simulink library "Renault_Library"  (Simulink 8.2)
settings: Solver=ode3  StartTime=0.0  StopTime=10.0  FixedStep=0.020  RelTol=1e-3
undefined symbols (not defined in the file or in its folder): ABC, AssystemProposal, b, chkval, CoeffA_C, CoeffB_C, CoeffMean_C, ConfTime_C, DiagDelay_C, DividePercent, FailureConfActive_C, FallingEdgeDuration_C, HighLevelThresholdVoltage_C, HighLevelVoltage_C, HighLevelVoltageTunable_C, InitValue_C, InputVoltRangeMax_C, InputVoltRangeMin_C, LowActiveContact_C, LowLevelThresholdVoltage_C, LowLevelVoltage_C, MaxOperatingVoltage_C, MinOperatingVoltage_C, mV2V_conversion, OUT1, OutOfRangeHigh_C, OutOfRangeLow_C, PinSupplySaturationMax, PinSupplySaturationMin, Res1Value_C, Res2Value_C, Res3Value_C, RisingEdgeDuration_C, RSA_CANSignalFailMonitoring, SampleTime_C, service14Table, service19Table, service22Table, service24Table, service28Table, service2ETable, service2FTable, service31Table, service87Table, SizeXStateDID2FTable, SizeXTable14, SizeXTable19, SizeXTable22, SizeXTable24, SizeXTable28, SizeXTable2E, SizeXTable2F, SizeXTable31, SizeXTable87, SizeYStateDID2FTable, SizeYTable14, SizeYTable19, SizeYTable22, SizeYTable24, SizeYTable28 ... (+22)
undefined symbols (not defined in the file): BatteryVoltage_ActivationThreshold_C, FrameStatus_AbsentValue_C, FrameStatus_ConfAbsentValue_C, MemThreshold_C, NAMEOFTHEMODEL_SAMPLE_TIME_CST, NbAbs1_C, NbConfAbs1_C, NbConfPres1_C, NbPres1_C, NOMDUSIGNAL_FaultConfTime_C, NOMDUSIGNAL_FaultRehabTime_C, NOMDUSIGNAL_switchvalue_C, NOMDUSIGNAL_unavailablevalue_C, PeriodFrame1_C, ResetTime_C, WakeUpTime_C
libraries: Renault_Library x112, simulink x75
(part 15 of 32)

== system Renault_Library/Services ECU/Commonly_Used_Blocks/RSA_ServECU_EnaOutContactSat/SwitchWithoutSlope ==
  In1 : Inport
  Switch_Cmd : Inport  Port=2
  In0 : Inport  Port=3
  Enable : EnablePort  StatesWhenEnabling=reset
  Switch3 : Switch  Criteria=u2 ~= 0  RndMeth=Zero  InputSameDT=on
  Out1 : Outport  OutputWhenDisabled=reset  InitialOutput=0
  wires:
  Switch_Cmd/1 -> Switch3/2
  In1/1 -> Switch3/1
  Switch3/1 -> Out1/1
  In0/1 -> Switch3/3

== system Renault_Library/Services ECU/Commonly_Used_Blocks/RSA_ServECU_EnabledDiagnostic/DiagDelaySubsystem ==
  In1 : Inport
  DataTypeConversion1 : DataTypeConversion  RndMeth=Zero
      settings: AttributesFormatString=Saturation:%<SaturateOnIntegerOverflow>
  DiagDelay : Constant  Value=DiagDelay_C  OutDataTypeStr=Inherit: Inherit via back propagation  SampleTime=-1
  RSA_TurnOffDelay2 : Renault_Library/Complex blocks/Timers & Counters/RSA_TurnOffDelay2  Tag=[LIB]  Ts=SampleTime_C
  RSA_TurnOnDelay2 : Renault_Library/Complex blocks/Timers & Counters/RSA_TurnOnDelay2  Tag=[LIB]  Ts=SampleTime_C
  Terminator1 : Terminator
  Terminator2 : Terminator
  ms2m : Gain  Gain=.001  RndMeth=Zero
      settings: AttributesFormatString=Saturation:%<SaturateOnIntegerOverflow>
  Out1 : Outport
  wires:
  RSA_TurnOnDelay2/1 -> RSA_TurnOffDelay2/1
  DiagDelay/1 -> ms2m/1
  RSA_TurnOnDelay2/2 -> Terminator1/1
  In1/1 -> DataTypeConversion1/1
  RSA_TurnOffDelay2/1 -> Out1/1
  RSA_TurnOffDelay2/2 -> Terminator2/1
  DataTypeConversion1/1 -> RSA_TurnOnDelay2/1
  ms2m/1 -> RSA_TurnOffDelay2/2, RSA_TurnOnDelay2/2

== system Renault_Library/Services ECU/Commonly_Used_Blocks/RSA_ServECU_EnabledSupply/Mean ==
  Output : Inport
  CoeffB2 : Constant  Value=CoeffMean_C  OutDataTypeStr=Inherit: Inherit via back propagation  SampleTime=-1
  Moy_1 : Subsystem
  Moy_10 : Subsystem
  Moy_2 : Subsystem
  Moy_3 : Subsystem
  Moy_4 : Subsystem
  Moy_5 : Subsystem
  Moy_6 : Subsystem
  Moy_7 : Subsystem
  Moy_8 : Subsystem
  Moy_9 : Subsystem
  MultiportSwitch : MultiPortSwitch  Inputs=10  RndMeth=Zero  InputSameDT=on
  MeanOutputVoltage : Outport
  wires:
  Moy_1/1(Out1) -> MultiportSwitch/2
  Moy_2/1(Out1) -> MultiportSwitch/3
  Moy_3/1(Out1) -> MultiportSwitch/4
  Moy_4/1(Out1) -> MultiportSwitch/5
  Moy_5/1(Out1) -> MultiportSwitch/6
  Moy_6/1(Out1) -> MultiportSwitch/7
  Moy_7/1(Out1) -> MultiportSwitch/8
  Moy_8/1(Out1) -> MultiportSwitch/9
  Moy_9/1(Out1) -> MultiportSwitch/10
  Moy_10/1(Out1) -> MultiportSwitch/11
  MultiportSwitch/1 -> MeanOutputVoltage/1
  CoeffB2/1 -> MultiportSwitch/1
  Output/1 -> Moy_1/1(In1), Moy_2/1(In1), Moy_3/1(In1), Moy_4/1(In1), Moy_5/1(In1), Moy_6/1(In1), Moy_7/1(In1), Moy_8/1(In1), Moy_9/1(In1), Moy_10/1(In1)

== system Renault_Library/Services ECU/Commonly_Used_Blocks/RSA_ServECU_FaultConfirmation/FailureMonitoring ==
  CurrentFaultState : Inport
  Failure : Inport  Port=2
  ConfTime : Constant  Value=ConfTime_C  OutDataTypeStr=Inherit: Inherit via back propagation  SampleTime=-1
  FixPtSum2 : Sum  OutDataTypeStr=Inherit: Inherit via back propagation  RndMeth=Zero  InputSameDT=on  LockScale=on
  FixPtSwitch2 : Switch  Criteria=u2 ~= 0  Threshold=0.5  OutDataTypeStr=Inherit: Inherit via back propagation  RndMeth=Zero  ZeroCross=off  InputSameDT=on
  Gain : Gain  Gain=.001  RndMeth=Zero
      settings: AttributesFormatString=Saturation:%<SaturateOnIntegerOverflow>
  Gain2 : Gain  Gain=0.001  RndMeth=Zero
      settings: AttributesFormatString=Saturation:%<SaturateOnIntegerOverflow>
  LogicalOperator1 : Logic  OutDataTypeStr=boolean
  Output1 : UnitDelay  SampleTime=-1
  RelationalOperator : RelationalOperator  Operator===  OutDataTypeStr=boolean  InputSameDT=on
  RelationalOperator1 : RelationalOperator  OutDataTypeStr=boolean  ZeroCross=off  InputSameDT=on
  Reset : Constant  Value=0  OutDataTypeStr=Inherit: Inherit via back propagation  SampleTime=-1
  SampleTime : Constant  Value=SampleTime_C  OutDataTypeStr=Inherit: Inherit via back propagation  SampleTime=-1
  Switch1 : Switch  Criteria=u2 ~= 0  Threshold=0.5  OutDataTypeStr=Inherit: Inherit via back propagation  RndMeth=Zero  SaturateOnIntegerOverflow=on  InputSameDT=on
  Switch4 : Switch  Criteria=u2 ~= 0  Threshold=0.5  RndMeth=Zero  InputSameDT=on
  UnitDelay : UnitDelay  SampleTime=-1
  UnitDelay1 : UnitDelay  InitialCondition=InitValue_C  SampleTime=-1
  false : Constant  Value=false  OutDataTypeStr=boolean  SampleTime=-1
  true : Constant  Value=true  OutDataTypeStr=boolean  SampleTime=-1
  ConfirmedFaultValue : Outport
  failure_flag : Outport  Port=2
  wires:
  LogicalOperator1/1 -> FixPtSwitch2/2
  RelationalOperator/1 -> LogicalOperator1/2
  [CurrentFaultState] -> RelationalOperator/1, UnitDelay/1
  UnitDelay/1 -> RelationalOperator/2
  Gain2/1 -> [SampleTime]
  Output1/1 -> FixPtSum2/1
  [SampleTime] -> FixPtSum2/2
  SampleTime/1 -> Gain2/1
  Reset/1 -> [Reset]
  CurrentFaultState/1 -> [CurrentFaultState], Switch1/1
  true/1 -> Switch4/1
  false/1 -> Switch4/3
  Switch4/1 -> Switch1/2, failure_flag/1
  [Reset] -> FixPtSwitch2/3
  Failure/1 -> LogicalOperator1/1
  FixPtSwitch2/1 -> RelationalOperator1/1, Output1/1
  FixPtSum2/1 -> FixPtSwitch2/1
  RelationalOperator1/1 -> Switch4/2
  UnitDelay1/1 -> Switch1/3
  Switch1/1 -> UnitDelay1/1, ConfirmedFaultValue/1
  ConfTime/1 -> Gain/1
  Gain/1 -> RelationalOperator1/2

== system Renault_Library/Services ECU/Diag/RSA_DiagnosticManagement/DIAGNOSTIC_ACTION_TOP ==
  DIAG_BUFFER_REQUEST : Inport  OutDataTypeStr=uint8  PortDimensions=4095
  NEGATIVE_RES_CODE : Inport  Port=2
  SUPPRESS_POS_RES : Inport  Port=3
  SEND_RES_MESSAGE : Inport  Port=4
  LIST_DID_SERVICE_22 : Inport  Port=5
  DIAG_INPUT : Inport  Port=6
  Diagnostic_Action : Stateflow chart  TreatAsAtomicUnit=on
  UnitDelay4 : UnitDelay  SampleTime=-1
  DIAG_OUTPUT : Outport
  DIAG_BUFFER_RESPONSE : Outport  Port=2  OutDataTypeStr=uint8  PortDimensions=4095
  RES_DATA_NUMBER : Outport  Port=3
  wires:
  SEND_RES_MESSAGE/1 -> Diagnostic_Action/4(SEND_RES_MESSAGE)
  NEGATIVE_RES_CODE/1 -> Diagnostic_Action/2(NEGATIVE_RES_CODE)
  LIST_DID_SERVICE_22/1 -> Diagnostic_Action/5(LIST_DID_SERVICE_22)
  Diagnostic_Action/2(DIAG_BUFFER_RESPONSE) -> DIAG_BUFFER_RESPONSE/1
  Diagnostic_Action/3(RES_DATA_NUMBER) -> UnitDelay4/1
  DIAG_BUFFER_REQUEST/1 -> Diagnostic_Action/1(DIAG_BUFFER_REQUEST)
  SUPPRESS_POS_RES/1 -> Diagnostic_Action/3(SUPPRESS_POS_RES)
  DIAG_INPUT/1 -> Diagnostic_Action/6(DIAG_INPUT)
  Diagnostic_Action/1(DIAG_OUTPUT) -> DIAG_OUTPUT/1
  UnitDelay4/1 -> RES_DATA_NUMBER/1

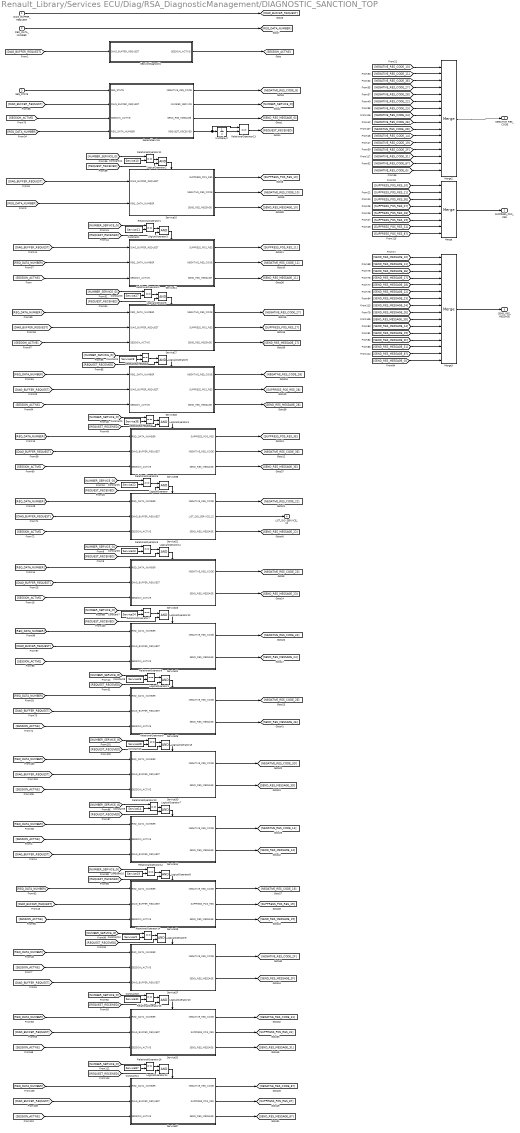
== system Renault_Library/Services ECU/Diag/RSA_DiagnosticManagement/DIAGNOSTIC_SANCTION_TOP ==
  DIAG_BUFFER_REQUEST : Inport  OutDataTypeStr=uint8  PortDimensions=4095
  REQ_DATA_NUMBER : Inport  Port=2  OutDataTypeStr=uint16
  SEG_STATE : Inport  Port=3  OutDataTypeStr=Enum: TYP_SEG_STATE
  Constant1 : Constant  Value=Service11  OutDataTypeStr=Inherit: Inherit via back propagation  SampleTime=-1
  Constant10 : Constant  Value=Service19  OutDataTypeStr=Inherit: Inherit via back propagation  SampleTime=-1
  Constant11 : Constant  Value=Service10  OutDataTypeStr=Inherit: Inherit via back propagation  SampleTime=-1
  Constant12 : Constant  Value=Service2F  OutDataTypeStr=Inherit: Inherit via back propagation  SampleTime=-1
  Constant13 : Constant  Value=Service3D  OutDataTypeStr=Inherit: Inherit via back propagation  SampleTime=-1
  Constant14 : Constant  Value=Service87  OutDataTypeStr=Inherit: Inherit via back propagation  SampleTime=-1
  Constant2 : Constant  Value=Service3E  OutDataTypeStr=Inherit: Inherit via back propagation  SampleTime=-1
  Constant20 : Constant  Value=Service31  OutDataTypeStr=Inherit: Inherit via back propagation  SampleTime=-1
  Constant3 : Constant  Value=Service27  OutDataTypeStr=Inherit: Inherit via back propagation  SampleTime=-1
  Constant4 : Constant  Value=Service28  OutDataTypeStr=Inherit: Inherit via back propagation  SampleTime=-1
  Constant5 : Constant  Value=Service22  OutDataTypeStr=Inherit: Inherit via back propagation  SampleTime=-1
  Constant6 : Constant  Value=Service23  OutDataTypeStr=Inherit: Inherit via back propagation  SampleTime=-1
  Constant7 : Constant  Value=Service24  OutDataTypeStr=Inherit: Inherit via back propagation  SampleTime=-1
  Constant8 : Constant  Value=Service2E  OutDataTypeStr=Inherit: Inherit via back propagation  SampleTime=-1
  Constant9 : Constant  Value=Service14  OutDataTypeStr=Inherit: Inherit via back propagation  SampleTime=-1
  GeneralService : Subsystem
  LogicalOperator{,1..14} (15 alike) : Logic  OutDataTypeStr=boolean
  Merge : Merge  Inputs=8
  Merge2 : Merge  Inputs=16
  Merge3 : Merge  Inputs=16
  RelationalOperator{1..16} (16 alike) : RelationalOperator  Operator===  OutDataTypeStr=boolean  InputSameDT=on
  Service10 : Subsystem  TreatAsAtomicUnit=on
  Service11 : Subsystem  TreatAsAtomicUnit=on
  Service14 : Subsystem  TreatAsAtomicUnit=on
  Service19 : Subsystem  TreatAsAtomicUnit=on
  Service22 : Subsystem  TreatAsAtomicUnit=on
  Service23 : Subsystem  TreatAsAtomicUnit=on
  Service24 : Subsystem  TreatAsAtomicUnit=on
  Service27 : Subsystem  TreatAsAtomicUnit=on
  Service28 : Subsystem  TreatAsAtomicUnit=on
  Service2E : Subsystem  TreatAsAtomicUnit=on
  Service2F : Subsystem  TreatAsAtomicUnit=on
  Service31 : Subsystem  TreatAsAtomicUnit=on
  Service3D : Subsystem  TreatAsAtomicUnit=on
  Service3E : Subsystem  TreatAsAtomicUnit=on
  Service87 : Subsystem  TreatAsAtomicUnit=on
  SessionDiagnostic : Subsystem
  UnitDelay2 : UnitDelay  SampleTime=-1
  NEGATIVE_RES_CODE : Outport
  SUPPRESS_POS_RES : Outport  Port=2
  SEND_RES_MESSAGE : Outport  Port=3
  LIST_DID_SERVICE_22 : Outport  Port=4  LockScale=on
  wires:
  [SESSION_ACTIVE] -> Service11/3(SESSION_ACTIVE)
  DIAG_BUFFER_REQUEST/1 -> [DIAG_BUFFER_REQUEST]
  REQ_DATA_NUMBER/1 -> [REQ_DATA_NUMBER]
  [DIAG_BUFFER_REQUEST] -> Service11/1(DIAG_BUFFER_REQUEST)
  [REQ_DATA_NUMBER] -> Service11/2(REQ_DATA_NUMBER)
  [REQ_DATA_NUMBER] -> Service14/1(REQ_DATA_NUMBER)
  SEG_STATE/1 -> GeneralService/1(SEG_STATE)
  [DIAG_BUFFER_REQUEST] -> GeneralService/2(DIAG_BUFFER_REQUEST)
  [SESSION_ACTIVE] -> GeneralService/3(SESSION_ACTIVE)
  GeneralService/2(NUMBER_SERVICE) -> [NUMBER_SERVICE_G]
  [NUMBER_SERVICE_G] -> RelationalOperator8/1
  Constant8/1 -> RelationalOperator8/2
  [REQ_DATA_NUMBER] -> Service2E/1(REQ_DATA_NUMBER)
  [REQ_DATA_NUMBER] -> Service19/1(REQ_DATA_NUMBER)
  [DIAG_BUFFER_REQUEST] -> Service19/2(DIAG_BUFFER_REQUEST)
  [SESSION_ACTIVE] -> Service19/3(SESSION_ACTIVE)
  [REQ_DATA_NUMBER] -> Service2F/1(REQ_DATA_NUMBER)
  [REQ_DATA_NUMBER] -> Service31/1(REQ_DATA_NUMBER)
  [DIAG_BUFFER_REQUEST] -> Service31/2(DIAG_BUFFER_REQUEST)
  [SESSION_ACTIVE] -> Service31/3(SESSION_ACTIVE)
  [DIAG_BUFFER_REQUEST] -> Service10/1(DIAG_BUFFER_REQUEST)
  [REQ_DATA_NUMBER] -> Service10/2(REQ_DATA_NUMBER)
  [DIAG_BUFFER_REQUEST] -> Service3E/2(DIAG_BUFFER_REQUEST)
  [REQ_DATA_NUMBER] -> Service3E/1(REQ_DATA_NUMBER)
  [SESSION_ACTIVE] -> Service3E/3(SESSION_ACTIVE)
  SessionDiagnostic/1(SESSION_ACTIVE) -> [SESSION_ACTIVE]
  [DIAG_BUFFER_REQUEST] -> SessionDiagnostic/1(DIAG_BUFFER_REQUEST)
  GeneralService/1(NEGATIVE_RES_CODE) -> [NEGATIVE_RES_CODE_G]
  Service10/1(SUPPRESS_POS_RES) -> [SUPPRESS_POS_RES_10]
  Service10/2(NEGATIVE_RES_CODE) -> [NEGATIVE_RES_CODE_10]
  Service11/1(SUPPRESS_POS_RES) -> [SUPPRESS_POS_RES_11]
  Service11/2(NEGATIVE_RES_CODE) -> [NEGATIVE_RES_CODE_11]
  Service3E/1(SUPPRESS_POS_RES) -> [SUPPRESS_POS_RES_3E]
  Service3E/2(NEGATIVE_RES_CODE) -> [NEGATIVE_RES_CODE_3E]
  Service2E/1(NEGATIVE_RES_CODE) -> [NEGATIVE_RES_CODE_2E]
  Service14/1(NEGATIVE_RES_CODE) -> [NEGATIVE_RES_CODE_14]
  Service19/1(NEGATIVE_RES_CODE) -> [NEGATIVE_RES_CODE_19]
  Service19/2(SUPPRESS_POS_RES) -> [SUPPRESS_POS_RES_19]
  Service2F/1(NEGATIVE_RES_CODE) -> [NEGATIVE_RES_CODE_2F]
  Service31/1(NEGATIVE_RES_CODE) -> [NEGATIVE_RES_CODE_31]
  Service31/2(SUPPRESS_POS_RES) -> [SUPPRESS_POS_RES_31]
  [DIAG_BUFFER_REQUEST] -> Service2E/2(DIAG_BUFFER_REQUEST)
  [SESSION_ACTIVE] -> Service2E/3(SESSION_ACTIVE)
  Service11/3(SEND_RES_MESSAGE) -> [SEND_RES_MESSAGE_11]
  Service3E/3(SEND_RES_MESSAGE) -> [SEND_RES_MESSAGE_3E]
  Service10/3(SEND_RES_MESSAGE) -> [SEND_RES_MESSAGE_10]
  Service2E/2(SEND_RES_MESSAGE) -> [SEND_RES_MESSAGE_2E]
  Service14/2(SEND_RES_MESSAGE) -> [SEND_RES_MESSAGE_14]
  Service19/3(SEND_RES_MESSAGE) -> [SEND_RES_MESSAGE_19]
  Service2F/2(SEND_RES_MESSAGE) -> [SEND_RES_MESSAGE_2F]
  Service31/3(SEND_RES_MESSAGE) -> [SEND_RES_MESSAGE_31]
  GeneralService/3(SEND_RES_MESSAGE) -> [SEND_RES_MESSAGE_G]
  UnitDelay2/1 -> RelationalOperator13/2
  RelationalOperator13/1 -> [REQUEST_RECEIVED]
  RelationalOperator8/1 -> LogicalOperator1/1
  [REQUEST_RECEIVED] -> LogicalOperator1/2
  LogicalOperator1/1 -> Service2E/Trigger
  [REQ_DATA_NUMBER] -> GeneralService/4(REQ_DATA_NUMBER)
  [NUMBER_SERVICE_G] -> RelationalOperator11/1
  Constant11/1 -> RelationalOperator11/2
  [REQUEST_RECEIVED] -> LogicalOperator2/2
  RelationalOperator11/1 -> LogicalOperator2/1
  [NUMBER_SERVICE_G] -> RelationalOperator1/1
  Constant1/1 -> RelationalOperator1/2
  [REQUEST_RECEIVED] -> LogicalOperator3/2
  RelationalOperator1/1 -> LogicalOperator3/1
  [NUMBER_SERVICE_G] -> RelationalOperator4/1
  Constant2/1 -> RelationalOperator4/2
  [REQUEST_RECEIVED] -> LogicalOperator4/2
  RelationalOperator4/1 -> LogicalOperator4/1
  [NUMBER_SERVICE_G] -> RelationalOperator10/1
  Constant9/1 -> RelationalOperator10/2
  [REQUEST_RECEIVED] -> LogicalOperator7/2
  RelationalOperator10/1 -> LogicalOperator7/1
  [NUMBER_SERVICE_G] -> RelationalOperator12/1
  Constant10/1 -> RelationalOperator12/2
  [REQUEST_RECEIVED] -> LogicalOperator8/2
  RelationalOperator12/1 -> LogicalOperator8/1
  [NUMBER_SERVICE_G] -> RelationalOperator14/1
  Constant12/1 -> RelationalOperator14/2
  [REQUEST_RECEIVED] -> LogicalOperator9/2
  RelationalOperator14/1 -> LogicalOperator9/1
  [NUMBER_SERVICE_G] -> RelationalOperator15/1
  Constant20/1 -> RelationalOperator15/2
  RelationalOperator15/1 -> LogicalOperator10/1
  LogicalOperator4/1 -> Service3E/Trigger
  LogicalOperator3/1 -> Service11/Trigger
  LogicalOperator2/1 -> Service10/Trigger
  LogicalOperator7/1 -> Service14/Trigger
  LogicalOperator8/1 -> Service19/Trigger
  LogicalOperator9/1 -> Service2F/Trigger
  LogicalOperator10/1 -> Service31/Trigger
  [SESSION_ACTIVE] -> Service14/2(SESSION_ACTIVE)
  [DIAG_BUFFER_REQUEST] -> Service14/3(DIAG_BUFFER_REQUEST)
  [SESSION_ACTIVE] -> Service2F/2(SESSION_ACTIVE)
  [DIAG_BUFFER_REQUEST] -> Service2F/3(DIAG_BUFFER_REQUEST)
  [REQ_DATA_NUMBER] -> Service22/1(REQ_DATA_NUMBER)
  Service22/1(NEGATIVE_RES_CODE) -> [NEGATIVE_RES_CODE_22]
  Service22/2(LIST_DID_SERVICE_22) -> LIST_DID_SERVICE_22/1
  [DIAG_BUFFER_REQUEST] -> Service22/2(DIAG_BUFFER_REQUEST)
  [SESSION_ACTIVE] -> Service22/3(SESSION_ACTIVE)
  Service22/3(SEND_RES_MESSAGE) -> [SEND_RES_MESSAGE_22]
  [NUMBER_SERVICE_G] -> RelationalOperator5/1
  Constant5/1 -> RelationalOperator5/2
  [REQUEST_RECEIVED] -> LogicalOperator/2
  RelationalOperator5/1 -> LogicalOperator/1
  LogicalOperator/1 -> Service22/Trigger
  [REQ_DATA_NUMBER] -> Service23/1(REQ_DATA_NUMBER)
  Service23/1(NEGATIVE_RES_CODE) -> [NEGATIVE_RES_CODE_23]
  [DIAG_BUFFER_REQUEST] -> Service23/2(DIAG_BUFFER_REQUEST)
  [SESSION_ACTIVE] -> Service23/3(SESSION_ACTIVE)
  Service23/2(SEND_RES_MESSAGE) -> [SEND_RES_MESSAGE_23]
  [NUMBER_SERVICE_G] -> RelationalOperator6/1
  Constant6/1 -> RelationalOperator6/2
  [REQUEST_RECEIVED] -> LogicalOperator11/2
  RelationalOperator6/1 -> LogicalOperator11/1
  LogicalOperator11/1 -> Service23/Trigger
  [REQ_DATA_NUMBER] -> Service24/1(REQ_DATA_NUMBER)
  Service24/1(NEGATIVE_RES_CODE) -> [NEGATIVE_RES_CODE_24]
  [DIAG_BUFFER_REQUEST] -> Service24/2(DIAG_BUFFER_REQUEST)
  [SESSION_ACTIVE] -> Service24/3(SESSION_ACTIVE)
  Service24/2(SEND_RES_MESSAGE) -> [SEND_RES_MESSAGE_24]
  [NUMBER_SERVICE_G] -> RelationalOperator7/1
  Constant7/1 -> RelationalOperator7/2
  [REQUEST_RECEIVED] -> LogicalOperator12/2
  RelationalOperator7/1 -> LogicalOperator12/1
  LogicalOperator12/1 -> Service24/Trigger
  [NUMBER_SERVICE_G] -> RelationalOperator9/1
  Constant13/1 -> RelationalOperator9/2
  [REQ_DATA_NUMBER] -> Service3D/1(REQ_DATA_NUMBER)
  Service3D/1(NEGATIVE_RES_CODE) -> [NEGATIVE_RES_CODE_3D]
  [DIAG_BUFFER_REQUEST] -> Service3D/2(DIAG_BUFFER_REQUEST)
  [SESSION_ACTIVE] -> Service3D/3(SESSION_ACTIVE)
  Service3D/2(SEND_RES_MESSAGE) -> [SEND_RES_MESSAGE_3D]
  RelationalOperator9/1 -> LogicalOperator13/1
  [REQUEST_RECEIVED] -> LogicalOperator13/2
  LogicalOperator13/1 -> Service3D/Trigger
  [REQ_DATA_NUMBER] -> Service87/1(REQ_DATA_NUMBER)
  [DIAG_BUFFER_REQUEST] -> Service87/2(DIAG_BUFFER_REQUEST)
  [SESSION_ACTIVE] -> Service87/3(SESSION_ACTIVE)
  Service87/1(NEGATIVE_RES_CODE) -> [NEGATIVE_RES_CODE_87]
  Service87/2(SUPPRESS_POS_RES) -> [SUPPRESS_POS_RES_87]
  Service87/3(SEND_RES_MESSAGE) -> [SEND_RES_MESSAGE_87]
  [NUMBER_SERVICE_G] -> RelationalOperator16/1
  Constant14/1 -> RelationalOperator16/2
  [REQUEST_RECEIVED] -> LogicalOperator14/2
  RelationalOperator16/1 -> LogicalOperator14/1
  LogicalOperator14/1 -> Service87/Trigger
  [REQ_DATA_NUMBER] -> Service27/1(REQ_DATA_NUMBER)
  [DIAG_BUFFER_REQUEST] -> Service27/2(DIAG_BUFFER_REQUEST)
  [REQ_DATA_NUMBER] -> Service28/1(REQ_DATA_NUMBER)
  [DIAG_BUFFER_REQUEST] -> Service28/2(DIAG_BUFFER_REQUEST)
  [SESSION_ACTIVE] -> Service27/3(SESSION_ACTIVE)
  [SESSION_ACTIVE] -> Service28/3(SESSION_ACTIVE)
  Service27/1(NEGATIVE_RES_CODE) -> [NEGATIVE_RES_CODE_27]
  Service27/2(SUPPRESS_POS_RES) -> [SUPPRESS_POS_RES_27]
  Service28/1(NEGATIVE_RES_CODE) -> [NEGATIVE_RES_CODE_28]
  Service28/2(SUPPRESS_POS_RES) -> [SUPPRESS_POS_RES_28]
  Service27/3(SEND_RES_MESSAGE) -> [SEND_RES_MESSAGE_27]
  Service28/3(SEND_RES_MESSAGE) -> [SEND_RES_MESSAGE_28]
  [NUMBER_SERVICE_G] -> RelationalOperator2/1
  Constant3/1 -> RelationalOperator2/2
  [REQUEST_RECEIVED] -> LogicalOperator5/2
  RelationalOperator2/1 -> LogicalOperator5/1
  [NUMBER_SERVICE_G] -> RelationalOperator3/1
  Constant4/1 -> RelationalOperator3/2
  [REQUEST_RECEIVED] -> LogicalOperator6/2
  RelationalOperator3/1 -> LogicalOperator6/1
  LogicalOperator6/1 -> Service28/Trigger
  LogicalOperator5/1 -> Service27/Trigger
  GeneralService/4(REQUEST_RECEIVED) -> UnitDelay2/1, RelationalOperator13/1
  [SEND_RES_MESSAGE_10] -> Merge3/1
  [SEND_RES_MESSAGE_11] -> Merge3/2
  [SEND_RES_MESSAGE_3E] -> Merge3/3
  [SEND_RES_MESSAGE_27] -> Merge3/4
  [SEND_RES_MESSAGE_28] -> Merge3/5
  [SEND_RES_MESSAGE_22] -> Merge3/6
  [SEND_RES_MESSAGE_23] -> Merge3/7
  [SEND_RES_MESSAGE_24] -> Merge3/8
  [SEND_RES_MESSAGE_2E] -> Merge3/9
  [SEND_RES_MESSAGE_3D] -> Merge3/10
  [SEND_RES_MESSAGE_14] -> Merge3/11
  [SEND_RES_MESSAGE_19] -> Merge3/12
  [SEND_RES_MESSAGE_2F] -> Merge3/13
  [SEND_RES_MESSAGE_31] -> Merge3/14
  [SEND_RES_MESSAGE_87] -> Merge3/15
  [SEND_RES_MESSAGE_G] -> Merge3/16
  Merge3/1 -> SEND_RES_MESSAGE/1
  [SUPPRESS_POS_RES_10] -> Merge/1
  [SUPPRESS_POS_RES_11] -> Merge/2
  [SUPPRESS_POS_RES_3E] -> Merge/3
  [SUPPRESS_POS_RES_27] -> Merge/4
  [SUPPRESS_POS_RES_28] -> Merge/5
  [SUPPRESS_POS_RES_19] -> Merge/6
  [SUPPRESS_POS_RES_31] -> Merge/7
  [SUPPRESS_POS_RES_87] -> Merge/8
  Merge/1 -> SUPPRESS_POS_RES/1
  [NEGATIVE_RES_CODE_10] -> Merge2/1
  [NEGATIVE_RES_CODE_11] -> Merge2/2
  [NEGATIVE_RES_CODE_3E] -> Merge2/3
  [NEGATIVE_RES_CODE_27] -> Merge2/4
  [NEGATIVE_RES_CODE_28] -> Merge2/5
  [NEGATIVE_RES_CODE_22] -> Merge2/6
  [NEGATIVE_RES_CODE_23] -> Merge2/7
  [NEGATIVE_RES_CODE_24] -> Merge2/8
  [NEGATIVE_RES_CODE_2E] -> Merge2/9
  [NEGATIVE_RES_CODE_3D] -> Merge2/10
  [NEGATIVE_RES_CODE_14] -> Merge2/11
  [NEGATIVE_RES_CODE_19] -> Merge2/12
  [NEGATIVE_RES_CODE_2F] -> Merge2/13
  [NEGATIVE_RES_CODE_31] -> Merge2/14
  [NEGATIVE_RES_CODE_87] -> Merge2/15
  [NEGATIVE_RES_CODE_G] -> Merge2/16
  Merge2/1 -> NEGATIVE_RES_CODE/1
  [REQUEST_RECEIVED] -> LogicalOperator10/2

== system Renault_Library/Services ECU/Diag/RSA_DiagnosticManagement/DIAGNOSTIC_SEGMENTATION_TOP ==
  DTOOL_TO_ECU_FRAME_RECEIVED : Inport  OutDataTypeStr=uint8
  PART1_DTOOL_TO_ECU : Inport  Port=2  OutDataTypeStr=uint32
  PART2_DTOOL_TO_ECU : Inport  Port=3  OutDataTypeStr=uint32
  DIAG_BUFFER_RESPONSE : Inport  Port=4  PortDimensions=4095
  RES_DATA_NUMBER : Inport  Port=5  OutDataTypeStr=uint16
  BusCreator2 : BusCreator  Inputs=8
  BusSelector1 : BusSelector  OutputSignals=DTOOL_TO_ECU_PART1.DTOOL_TO_ECU_BYTE_0,DTOOL_TO_ECU_PART1.DTOOL_TO_ECU_BYTE_1,DTOOL_TO_ECU_PART1.DTOOL_TO_ECU_BYTE_2,DTOOL_TO_ECU_PART1.DTOOL_TO_ECU_BYTE_3,DTOOL_TO_ECU_PART2.DTOOL_TO_ECU_BYTE_4,DTOOL_TO_ECU_PART2.DTOOL_TO_ECU_BYTE_5,DTOOL_TO_ECU_PART2.DTOOL_TO_ECU_BYTE_6,DTOOL_TO_ECU_PART2.DTOOL_TO_ECU_BYTE_7
  Diagnostic_Segmentation : Stateflow chart  TreatAsAtomicUnit=on
  Frame_Type : Subsystem
  Request_Frame : Subsystem
  Response_Frame : Subsystem
  UnitDelay : UnitDelay  SampleTime=-1
  ECU_TO_DTOOL_SEND : Outport  OutDataTypeStr=uint8
  PART1_ECU_TO_DTOOL : Outport  Port=2  OutDataTypeStr=uint32
  PART2_ECU_TO_DTOOL : Outport  Port=3  OutDataTypeStr=uint32
  DIAG_BUFFER_REQUEST : Outport  Port=4  OutDataTypeStr=uint8  PortDimensions=4095
  REQ_DATA_NUMBER : Outport  Port=5  OutDataTypeStr=uint16
  SEG_STATE : Outport  Port=6
  wires:
  PART2_DTOOL_TO_ECU/1 -> Request_Frame/2(PART2_DTOOL_TO_ECU)
  Request_Frame/1(DTOOL_TO_ECU) -> BusSelector1/1, Frame_Type/1(DTOOL_TO_ECU)  "DTOOL_TO_ECU"
  BusSelector1/2 -> Diagnostic_Segmentation/4(DTOOL_TO_ECU_BYTE_1)  "<DTOOL_TO_ECU_BYTE_1>"
  BusSelector1/3 -> Diagnostic_Segmentation/5(DTOOL_TO_ECU_BYTE_2)  "<DTOOL_TO_ECU_BYTE_2>"
  BusSelector1/4 -> Diagnostic_Segmentation/6(DTOOL_TO_ECU_BYTE_3)  "<DTOOL_TO_ECU_BYTE_3>"
  BusSelector1/5 -> Diagnostic_Segmentation/7(DTOOL_TO_ECU_BYTE_4)  "<DTOOL_TO_ECU_BYTE_4>"
  BusSelector1/6 -> Diagnostic_Segmentation/8(DTOOL_TO_ECU_BYTE_5)  "<DTOOL_TO_ECU_BYTE_5>"
  BusSelector1/7 -> Diagnostic_Segmentation/9(DTOOL_TO_ECU_BYTE_6)  "<DTOOL_TO_ECU_BYTE_6>"
  BusSelector1/8 -> Diagnostic_Segmentation/10(DTOOL_TO_ECU_BYTE_7)  "<DTOOL_TO_ECU_BYTE_7>"
  Diagnostic_Segmentation/5(ECU_TO_DTOOL_BYTE_0) -> BusCreator2/1
  Diagnostic_Segmentation/6(ECU_TO_DTOOL_BYTE_1) -> BusCreator2/2
  Diagnostic_Segmentation/7(ECU_TO_DTOOL_BYTE_2) -> BusCreator2/3
  Diagnostic_Segmentation/8(ECU_TO_DTOOL_BYTE_3) -> BusCreator2/4
  Diagnostic_Segmentation/9(ECU_TO_DTOOL_BYTE_4) -> BusCreator2/5
  Diagnostic_Segmentation/10(ECU_TO_DTOOL_BYTE_5) -> BusCreator2/6
  Diagnostic_Segmentation/11(ECU_TO_DTOOL_BYTE_6) -> BusCreator2/7
  Diagnostic_Segmentation/12(ECU_TO_DTOOL_BYTE_7) -> BusCreator2/8
  DIAG_BUFFER_RESPONSE/1 -> UnitDelay/1
  RES_DATA_NUMBER/1 -> Diagnostic_Segmentation/12(RES_DATA_NUMBER)
  PART1_DTOOL_TO_ECU/1 -> Request_Frame/1(PART1_DTOOL_TO_ECU)
  BusSelector1/1 -> Diagnostic_Segmentation/3(DTOOL_TO_ECU_BYTE_0)  "<DTOOL_TO_ECU_BYTE_0>"
  Diagnostic_Segmentation/3(ECU_TO_DTOOL_SEND) -> ECU_TO_DTOOL_SEND/1
  Frame_Type/1(REQ_FRAME_TYPE) -> Diagnostic_Segmentation/2(REQ_FRAME_TYPE)
  Response_Frame/1(PART1_ECU_TO_DTOOL) -> PART1_ECU_TO_DTOOL/1